simulink model "pfb_fft_vacc_sfloat_fft_dev"  (R2016b) (continued)
== system pfb_fft_vacc_sfloat_fft_dev == (continued)
      mask init:
      | pipeline_init(gcb,'latency',latency);
      settings: AttributesFormatString=Latency = %<latency>
      mask Display:
      | port_label('input', 1, 'd');
      | port_label('output', 1, 'q');
      | disp(['Z^-', num2str(latency)]);
  pipeline87 : Subsystem  AncestorBlock=casper_library_delays/pipeline  mask(pipeline){latency=1}
      mask help:
      | An explicitly laid-out delay line to avoid mapping to SRL16s
      | for use in pipelining to help achieve timing closure.
      mask init:
      | pipeline_init(gcb,'latency',latency);
      settings: AttributesFormatString=Latency = %<latency>
      mask Display:
      | port_label('input', 1, 'd');
      | port_label('output', 1, 'q');
      | disp(['Z^-', num2str(latency)]);
  pipeline88 : Subsystem  AncestorBlock=casper_library_delays/pipeline  mask(pipeline){latency=1}
      mask help:
      | An explicitly laid-out delay line to avoid mapping to SRL16s
      | for use in pipelining to help achieve timing closure.
      mask init:
      | pipeline_init(gcb,'latency',latency);
      settings: AttributesFormatString=Latency = %<latency>
      mask Display:
      | port_label('input', 1, 'd');
      | port_label('output', 1, 'q');
      | disp(['Z^-', num2str(latency)]);
  pipeline89 : Subsystem  AncestorBlock=casper_library_delays/pipeline  mask(pipeline){latency=1}
      mask help:
      | An explicitly laid-out delay line to avoid mapping to SRL16s
      | for use in pipelining to help achieve timing closure.
      mask init:
      | pipeline_init(gcb,'latency',latency);
      settings: AttributesFormatString=Latency = %<latency>
      mask Display:
      | port_label('input', 1, 'd');
      | port_label('output', 1, 'q');
      | disp(['Z^-', num2str(latency)]);
  pipeline9 : Subsystem  AncestorBlock=casper_library_delays/pipeline  mask(pipeline){latency=2}
      mask help:
      | An explicitly laid-out delay line to avoid mapping to SRL16s
      | for use in pipelining to help achieve timing closure.
      mask init:
      | pipeline_init(gcb,'latency',latency);
      settings: AttributesFormatString=Latency = %<latency>
      mask Display:
      | port_label('input', 1, 'd');
      | port_label('output', 1, 'q');
      | disp(['Z^-', num2str(latency)]);
  pipeline90 : Subsystem  AncestorBlock=casper_library_delays/pipeline  mask(pipeline){latency=2}
      mask help:
      | An explicitly laid-out delay line to avoid mapping to SRL16s
      | for use in pipelining to help achieve timing closure.
      mask init:
      | pipeline_init(gcb,'latency',latency);
      settings: AttributesFormatString=Latency = %<latency>
      mask Display:
      | port_label('input', 1, 'd');
      | port_label('output', 1, 'q');
      | disp(['Z^-', num2str(latency)]);
  pipeline91 : Subsystem  AncestorBlock=casper_library_delays/pipeline  mask(pipeline){latency=1}
      mask help:
      | An explicitly laid-out delay line to avoid mapping to SRL16s
      | for use in pipelining to help achieve timing closure.
      mask init:
      | pipeline_init(gcb,'latency',latency);
      settings: AttributesFormatString=Latency = %<latency>
      mask Display:
      | port_label('input', 1, 'd');
      | port_label('output', 1, 'q');
      | disp(['Z^-', num2str(latency)]);
  pipeline92 : Subsystem  AncestorBlock=casper_library_delays/pipeline  mask(pipeline){latency=1}
      mask help:
      | An explicitly laid-out delay line to avoid mapping to SRL16s
      | for use in pipelining to help achieve timing closure.
      mask init:
      | pipeline_init(gcb,'latency',latency);
      settings: AttributesFormatString=Latency = %<latency>
      mask Display:
      | port_label('input', 1, 'd');
      | port_label('output', 1, 'q');
      | disp(['Z^-', num2str(latency)]);
  pipeline93 : Subsystem  AncestorBlock=casper_library_delays/pipeline  mask(pipeline){latency=1}
      mask help:
      | An explicitly laid-out delay line to avoid mapping to SRL16s
      | for use in pipelining to help achieve timing closure.
      mask init:
      | pipeline_init(gcb,'latency',latency);
      settings: AttributesFormatString=Latency = %<latency>
      mask Display:
      | port_label('input', 1, 'd');
      | port_label('output', 1, 'q');
      | disp(['Z^-', num2str(latency)]);
  pipeline94 : Subsystem  AncestorBlock=casper_library_delays/pipeline  mask(pipeline){latency=2}
      mask help:
      | An explicitly laid-out delay line to avoid mapping to SRL16s
      | for use in pipelining to help achieve timing closure.
      mask init:
      | pipeline_init(gcb,'latency',latency);
      settings: AttributesFormatString=Latency = %<latency>
      mask Display:
      | port_label('input', 1, 'd');
      | port_label('output', 1, 'q');
      | disp(['Z^-', num2str(latency)]);
  pipeline95 : Subsystem  AncestorBlock=casper_library_delays/pipeline  mask(pipeline){latency=1}
      mask help:
      | An explicitly laid-out delay line to avoid mapping to SRL16s
      | for use in pipelining to help achieve timing closure.
      mask init:
      | pipeline_init(gcb,'latency',latency);
      settings: AttributesFormatString=Latency = %<latency>
      mask Display:
      | port_label('input', 1, 'd');
      | port_label('output', 1, 'q');
      | disp(['Z^-', num2str(latency)]);
  pipeline96 : Subsystem  AncestorBlock=casper_library_delays/pipeline  mask(pipeline){latency=2}
      mask help:
      | An explicitly laid-out delay line to avoid mapping to SRL16s
      | for use in pipelining to help achieve timing closure.
      mask init:
      | pipeline_init(gcb,'latency',latency);
      settings: AttributesFormatString=Latency = %<latency>
      mask Display:
      | port_label('input', 1, 'd');
      | port_label('output', 1, 'q');
      | disp(['Z^-', num2str(latency)]);
  pipeline97 : Subsystem  AncestorBlock=casper_library_delays/pipeline  mask(pipeline){latency=4}
      mask help:
      | An explicitly laid-out delay line to avoid mapping to SRL16s
      | for use in pipelining to help achieve timing closure.
      mask init:
      | pipeline_init(gcb,'latency',latency);
      settings: AttributesFormatString=Latency = %<latency>
      mask Display:
      | port_label('input', 1, 'd');
      | port_label('output', 1, 'q');
      | disp(['Z^-', num2str(latency)]);
  pipeline98 : Subsystem  AncestorBlock=casper_library_delays/pipeline  mask(pipeline){latency=2}
      mask help:
      | An explicitly laid-out delay line to avoid mapping to SRL16s
      | for use in pipelining to help achieve timing closure.
      mask init:
      | pipeline_init(gcb,'latency',latency);
      settings: AttributesFormatString=Latency = %<latency>
      mask Display:
      | port_label('input', 1, 'd');
      | port_label('output', 1, 'q');
      | disp(['Z^-', num2str(latency)]);
  pipeline99 : Subsystem  AncestorBlock=casper_library_delays/pipeline  mask(pipeline){latency=1}
      mask help:
      | An explicitly laid-out delay line to avoid mapping to SRL16s
      | for use in pipelining to help achieve timing closure.
      mask init:
      | pipeline_init(gcb,'latency',latency);
      settings: AttributesFormatString=Latency = %<latency>
      mask Display:
      | port_label('input', 1, 'd');
      | port_label('output', 1, 'q');
      | disp(['Z^-', num2str(latency)]);
  real_square{,1..3} (4 alike) : xbsIndex_r4/Mult  precision=Full  arith_type="Signed  (2's comp)"  n_bits=8  bin_pt=2  quantization=Truncate  overflow=Wrap  en=off  latency=6  use_behavioral_HDL=off  opt=Speed  use_embedded=on  optimum_pipeline=off  pipeline=on  dbl_ovrd=off  use_rpm=on  placement_style=Rectangular shape  block_type=mult
      settings: xl_area=[0, 0, 0, 0, 0, 0, 0]  block_version=9.1.01
  rpt_en_cwg0 : Subsystem  Tag=xps:sw_reg  AncestorBlock=xps_library/software_register  mask(swreg){io_dir=From Processor, io_delay=1, sample_period=1, names=[en dummy], bitwidths=[1 31], bin_pts=[0 0], arith_types=[0 0], sim_port=on, show_format=on}
      mask prompts: io_dir "I/O direction"; io_delay "I/O delay"; sample_period "Sample period"; names "Bitfield names [msb...lsb]"; bitwidths "Bitfield widths"; bin_pts "Bitfield binary pts"; arith_types "Bitfield types, ufix=0, fix=1, bool=2"; sim_port "Provide sim input/output?"; show_format "Print format string?"
      mask help: A 32-bit software-accessible register. Can be divided into bitfields of varying widths and types using the fields in the block mask.
      mask init:
      | swreg_init(gcb)
      settings: AttributesFormatString=2 inputs:uf1.0,uf31.0 = 32 bits
      mask TypeOptions io_dir: To Processor | From Processor
      mask Callback io_dir:
      | mask_enables = get_param(gcb, 'MaskEnables');
      | mask_names = get_param(gcb, 'MaskNames');
      | if strcmp(get_param(gcb, 'io_dir'), 'To Processor'),
      |     mask_enables{ismember(mask_names, 'sample_period')} = 'off';
      | else
      |     mask_enables{ismember(mask_names, 'sample_period')} = 'on';
      | end
      | set_param(gcb, 'MaskEnables', mask_enables);
  rpt_length_cwg0 : Subsystem  Tag=xps:sw_reg  AncestorBlock=xps_library/software_register  mask(swreg){io_dir=From Processor, io_delay=1, sample_period=1, names=cwg0_repeat_length, bitwidths=32, bin_pts=0, arith_types=0, sim_port=on, show_format=on}
      mask prompts: io_dir "I/O direction"; io_delay "I/O delay"; sample_period "Sample period"; names "Bitfield names [msb...lsb]"; bitwidths "Bitfield widths"; bin_pts "Bitfield binary pts"; arith_types "Bitfield types, ufix=0, fix=1, bool=2"; sim_port "Provide sim input/output?"; show_format "Print format string?"
      mask help: A 32-bit software-accessible register. Can be divided into bitfields of varying widths and types using the fields in the block mask.
      mask init:
      | swreg_init(gcb)
      settings: AttributesFormatString=1input:uf32.0 = 32 bits
      mask TypeOptions io_dir: To Processor | From Processor
      mask Callback io_dir:
      | mask_enables = get_param(gcb, 'MaskEnables');
      | mask_names = get_param(gcb, 'MaskNames');
      | if strcmp(get_param(gcb, 'io_dir'), 'To Processor'),
      |     mask_enables{ismember(mask_names, 'sample_period')} = 'off';
      | else
      |     mask_enables{ismember(mask_names, 'sample_period')} = 'on';
      | end
      | set_param(gcb, 'MaskEnables', mask_enables);
  scale_cwg0 : Subsystem  Tag=xps:sw_reg  AncestorBlock=xps_library/software_register  mask(swreg){io_dir=From Processor, io_delay=1, sample_period=1, names=[scale dummy], bitwidths=16, bin_pts=15, arith_types=0, sim_port=on, show_format=on}
      mask prompts: io_dir "I/O direction"; io_delay "I/O delay"; sample_period "Sample period"; names "Bitfield names [msb...lsb]"; bitwidths "Bitfield widths"; bin_pts "Bitfield binary pts"; arith_types "Bitfield types, ufix=0, fix=1, bool=2"; sim_port "Provide sim input/output?"; show_format "Print format string?"
      mask help: A 32-bit software-accessible register. Can be divided into bitfields of varying widths and types using the fields in the block mask.
      mask init:
      | swreg_init(gcb)
      settings: AttributesFormatString=2 inputs:uf16.15,uf16.15 = 32 bits
      mask TypeOptions io_dir: To Processor | From Processor
      mask Callback io_dir:
      | mask_enables = get_param(gcb, 'MaskEnables');
      | mask_names = get_param(gcb, 'MaskNames');
      | if strcmp(get_param(gcb, 'io_dir'), 'To Processor'),
      |     mask_enables{ismember(mask_names, 'sample_period')} = 'off';
      | else
      |     mask_enables{ismember(mask_names, 'sample_period')} = 'on';
      | end
      | set_param(gcb, 'MaskEnables', mask_enables);
  scale_float : Subsystem
  scale_float1 : Subsystem
  scale_float2 : Subsystem
  scale_float3 : Subsystem
  scale_out0 : Subsystem  Tag=xps:sw_reg  AncestorBlock=xps_library/software_register  mask(swreg){io_dir=From Processor, io_delay=1, sample_period=1, names=[scale dummy], bitwidths=16, bin_pts=15, arith_types=0, sim_port=on, show_format=on}
      mask prompts: io_dir "I/O direction"; io_delay "I/O delay"; sample_period "Sample period"; names "Bitfield names [msb...lsb]"; bitwidths "Bitfield widths"; bin_pts "Bitfield binary pts"; arith_types "Bitfield types, ufix=0, fix=1, bool=2"; sim_port "Provide sim input/output?"; show_format "Print format string?"
      mask help: A 32-bit software-accessible register. Can be divided into bitfields of varying widths and types using the fields in the block mask.
      mask init:
      | swreg_init(gcb)
      settings: AttributesFormatString=2 inputs:uf16.15,uf16.15 = 32 bits
      mask TypeOptions io_dir: To Processor | From Processor
      mask Callback io_dir:
      | mask_enables = get_param(gcb, 'MaskEnables');
      | mask_names = get_param(gcb, 'MaskNames');
      | if strcmp(get_param(gcb, 'io_dir'), 'To Processor'),
      |     mask_enables{ismember(mask_names, 'sample_period')} = 'off';
      | else
      |     mask_enables{ismember(mask_names, 'sample_period')} = 'on';
      | end
      | set_param(gcb, 'MaskEnables', mask_enables);
  scale_wng0 : Subsystem  Tag=xps:sw_reg  AncestorBlock=xps_library/software_register  mask(swreg){io_dir=From Processor, io_delay=1, sample_period=1, names=[scale dummy], bitwidths=16, bin_pts=15, arith_types=0, sim_port=on, show_format=on}
      mask prompts: io_dir "I/O direction"; io_delay "I/O delay"; sample_period "Sample period"; names "Bitfield names [msb...lsb]"; bitwidths "Bitfield widths"; bin_pts "Bitfield binary pts"; arith_types "Bitfield types, ufix=0, fix=1, bool=2"; sim_port "Provide sim input/output?"; show_format "Print format string?"
      mask help: A 32-bit software-accessible register. Can be divided into bitfields of varying widths and types using the fields in the block mask.
      mask init:
      | swreg_init(gcb)
      settings: AttributesFormatString=2 inputs:uf16.15,uf16.15 = 32 bits
      mask TypeOptions io_dir: To Processor | From Processor
      mask Callback io_dir:
      | mask_enables = get_param(gcb, 'MaskEnables');
      | mask_names = get_param(gcb, 'MaskNames');
      | if strcmp(get_param(gcb, 'io_dir'), 'To Processor'),
      |     mask_enables{ismember(mask_names, 'sample_period')} = 'off';
      | else
      |     mask_enables{ismember(mask_names, 'sample_period')} = 'on';
      | end
      | set_param(gcb, 'MaskEnables', mask_enables);
  simple_bram_vacc : Subsystem  AncestorBlock=casper_library_accumulators/simple_bram_vacc  mask(simple_bram_vacc){vec_len=2^10, arith_type=Signed, n_bits=32, bin_pt=7}
      mask prompts: vec_len "Vector length:"; arith_type "Output type:"; n_bits "Bit width (output):"; bin_pt "Binary point (output):"
      mask help: This simple vector accumulator is based on a shift register. It outputs its previous accumulated vector after a new_acc pulse is received.
      mask init:
      | simple_bram_vacc_init(gcb, ...
      |     'vec_len', vec_len, ...
      |     'arith_type', arith_type, ...
      |     'n_bits', n_bits, ...
      |     'bin_pt', bin_pt);
      mask TypeOptions arith_type: Signed | Unsigned
  simple_bram_vacc1 : Subsystem  AncestorBlock=casper_library_accumulators/simple_bram_vacc  mask(simple_bram_vacc){vec_len=2^10, arith_type=Signed, n_bits=32, bin_pt=7}
      mask prompts: vec_len "Vector length:"; arith_type "Output type:"; n_bits "Bit width (output):"; bin_pt "Binary point (output):"
      mask help: This simple vector accumulator is based on a shift register. It outputs its previous accumulated vector after a new_acc pulse is received.
      mask init:
      | simple_bram_vacc_init(gcb, ...
      |     'vec_len', vec_len, ...
      |     'arith_type', arith_type, ...
      |     'n_bits', n_bits, ...
      |     'bin_pt', bin_pt);
      mask TypeOptions arith_type: Signed | Unsigned
  simple_bram_vacc2 : Subsystem  AncestorBlock=casper_library_accumulators/simple_bram_vacc  mask(simple_bram_vacc){vec_len=2^10, arith_type=Signed, n_bits=32, bin_pt=7}
      mask prompts: vec_len "Vector length:"; arith_type "Output type:"; n_bits "Bit width (output):"; bin_pt "Binary point (output):"
      mask help: This simple vector accumulator is based on a shift register. It outputs its previous accumulated vector after a new_acc pulse is received.
      mask init:
      | simple_bram_vacc_init(gcb, ...
      |     'vec_len', vec_len, ...
      |     'arith_type', arith_type, ...
      |     'n_bits', n_bits, ...
      |     'bin_pt', bin_pt);
      mask TypeOptions arith_type: Signed | Unsigned
  simple_bram_vacc3 : Subsystem  AncestorBlock=casper_library_accumulators/simple_bram_vacc  mask(simple_bram_vacc){vec_len=2^10, arith_type=Signed, n_bits=32, bin_pt=7}
      mask prompts: vec_len "Vector length:"; arith_type "Output type:"; n_bits "Bit width (output):"; bin_pt "Binary point (output):"
      mask help: This simple vector accumulator is based on a shift register. It outputs its previous accumulated vector after a new_acc pulse is received.
      mask init:
      | simple_bram_vacc_init(gcb, ...
      |     'vec_len', vec_len, ...
      |     'arith_type', arith_type, ...
      |     'n_bits', n_bits, ...
      |     'bin_pt', bin_pt);
      mask TypeOptions arith_type: Signed | Unsigned
  simple_bram_vacc_float1 : Subsystem  AncestorBlock=casper_library_accumulators/simple_bram_vacc  mask(simple_bram_vacc){vec_len=2^10, arith_type=Unsigned, n_bits=128, bin_pt=68}
      mask prompts: vec_len "Vector length:"; arith_type "Output type:"; n_bits "Bit width (output):"; bin_pt "Binary point (output):"
      mask help: This simple vector accumulator is based on a shift register. It outputs its previous accumulated vector after a new_acc pulse is received.
      mask init:
      | % simple_bram_vacc_init(gcb, ...
      | %     'vec_len', vec_len, ...
      | %     'arith_type', arith_type, ...
      | %     'n_bits', n_bits, ...
      | %     'bin_pt', bin_pt);
      mask TypeOptions arith_type: Signed | Unsigned
  spect_cnt : xbsIndex_r4/Counter  cnt_type=Free Running  cnt_to=Inf  operation=Up  start_count=0  cnt_by_val=1  arith_type=Unsigned  n_bits=32  bin_pt=0  load_pin=off  rst=on  en=on  explicit_period=on  period=1  use_behavioral_HDL=off  implementation=Fabric  use_rpm=off  dbl_ovrd=off  block_type=counter
  spectrum_counter : Subsystem  Tag=xps:sw_reg  AncestorBlock=xps_library/software_register  mask(swreg){io_dir=To Processor, io_delay=0, sample_period=1, names=reg, bitwidths=32, bin_pts=0, arith_types=0, sim_port=on, show_format=on}
      mask prompts: io_dir "I/O direction"; io_delay "I/O delay"; sample_period "Sample period"; names "Bitfield names [msb...lsb]"; bitwidths "Bitfield widths"; bin_pts "Bitfield binary pts"; arith_types "Bitfield types, ufix=0, fix=1, bool=2"; sim_port "Provide sim input/output?"; show_format "Print format string?"
      mask help: A 32-bit software-accessible register. Can be divided into bitfields of varying widths and types using the fields in the block mask.
      mask init:
      | swreg_init(gcb)
      settings: AttributesFormatString=1output:uf32.0 = 32 bits
      mask TypeOptions io_dir: To Processor | From Processor
      mask Callback io_dir:
      | mask_enables = get_param(gcb, 'MaskEnables');
      | mask_names = get_param(gcb, 'MaskNames');
      | if strcmp(get_param(gcb, 'io_dir'), 'To Processor'),
      |     mask_enables{ismember(mask_names, 'sample_period')} = 'off';
      | else
      |     mask_enables{ismember(mask_names, 'sample_period')} = 'on';
      | end
      | set_param(gcb, 'MaskEnables', mask_enables);
  spectrum_limit : Subsystem  Tag=xps:sw_reg  AncestorBlock=xps_library/software_register  mask(swreg){io_dir=From Processor, io_delay=0, sample_period=1, names=limit, bitwidths=32, bin_pts=0, arith_types=0, sim_port=on, show_format=on}
      mask prompts: io_dir "I/O direction"; io_delay "I/O delay"; sample_period "Sample period"; names "Bitfield names [msb...lsb]"; bitwidths "Bitfield widths"; bin_pts "Bitfield binary pts"; arith_types "Bitfield types, ufix=0, fix=1, bool=2"; sim_port "Provide sim input/output?"; show_format "Print format string?"
      mask help: A 32-bit software-accessible register. Can be divided into bitfields of varying widths and types using the fields in the block mask.
      mask init:
      | swreg_init(gcb)
      settings: AttributesFormatString=1input:uf32.0 = 32 bits
      mask TypeOptions io_dir: To Processor | From Processor
      mask Callback io_dir:
      | mask_enables = get_param(gcb, 'MaskEnables');
      | mask_names = get_param(gcb, 'MaskNames');
      | if strcmp(get_param(gcb, 'io_dir'), 'To Processor'),
      |     mask_enables{ismember(mask_names, 'sample_period')} = 'off';
      | else
      |     mask_enables{ismember(mask_names, 'sample_period')} = 'on';
      | end
      | set_param(gcb, 'MaskEnables', mask_enables);
  ss_pfb_raw : Subsystem  Tag=casper:bitsnap  AncestorBlock=casper_library_scopes/bitfield_snapshot  mask(bitsnap){io_names=[r0 i0 r1 i1 acc], io_widths=[18 18 18 18 32], io_bps=[17 17 17 17 0], io_types=[1 1 1 1 0], show_format=on, snap_storage=bram, snap_dram_dimm=2, snap_dram_clock=250, snap_nsamples=10, snap_data_width=128, snap_offset=off, snap_circap=off, snap_value=off, snap_ext_arm=off, snap_provide_outputs=on, snap_use_dsp48=on, snap_delay=2, extra_names=[c d], extra_widths=[30 2], extra_bps=[0 0], extra_types=[0 0]}
      mask prompts: io_names "Names [msb...lsb]"; io_widths "Bitwidths [msb...lsb]"; io_bps "Binary pts [msb...lsb]"; io_types "Data types [msb...lsb], ufix=0, fix=1, bool=2"; show_format "Print format string?"; snap_storage "Storage medium"; snap_dram_dimm "DRAM dimm"; snap_dram_clock "DRAM clock rate"; snap_nsamples "Number of Samples (2^?)"; snap_data_width "Data width"; snap_offset "Start delay support"; snap_circap "Circular capture support"; snap_value "Extra value capture support"; snap_ext_arm "External arm support"; snap_provide_outputs "Provide outputs"; snap_use_dsp48 "Use DSP48s to implement counters"; snap_delay "Pre-snapshot delay"; extra_names "Names [msb...lsb]"; extra_widths "Widths [msb...lsb]"; extra_bps "Binary pts [msb...lsb]"; extra_types "Data types [msb...lsb], ufix=0, fix=1, bool=2"
      mask help:
      | A wrapped snapshot block. Allows specification of inputs to a snapblock word so the toolflow can automatically set up the scope.
      | See documentation for snapshot for explanation of parameters.
      mask init:
      | bitsnap_init(gcb);
      settings: AttributesFormatString=|
      | snap(5):f18.17,f18.17,f18.17,f18.17,uf32.0 = 104 bits\n128 wide, 1024 deep
      | debugID: 130880866
      mask TypeOptions snap_storage: bram | dram
      mask Callback snap_storage: bitsnap_callback();
      mask TypeOptions snap_dram_dimm: 1 | 2 | 3 | 4
      mask TypeOptions snap_dram_clock: 200 | 250 | 266 | 300 | 333
      mask TypeOptions snap_data_width: 8 | 16 | 32 | 64 | 128
      mask Callback snap_value: bitsnap_callback();
      mask Callback snap_ext_arm: bitsnap_callback();
      mask Callback snap_provide_outputs: bitsnap_callback();
  ss_pfb_raw1 : Subsystem  Tag=casper:bitsnap  AncestorBlock=casper_library_scopes/bitfield_snapshot  mask(bitsnap){io_names=[r2 i2 r3 i3], io_widths=[18 18 18 18], io_bps=[17 17 17 17], io_types=[1 1 1 1], show_format=on, snap_storage=bram, snap_dram_dimm=2, snap_dram_clock=250, snap_nsamples=10, snap_data_width=128, snap_offset=off, snap_circap=off, snap_value=off, snap_ext_arm=off, snap_provide_outputs=on, snap_use_dsp48=on, snap_delay=2, extra_names=[c d], extra_widths=[30 2], extra_bps=[0 0], extra_types=[0 0]}
      mask prompts: io_names "Names [msb...lsb]"; io_widths "Bitwidths [msb...lsb]"; io_bps "Binary pts [msb...lsb]"; io_types "Data types [msb...lsb], ufix=0, fix=1, bool=2"; show_format "Print format string?"; snap_storage "Storage medium"; snap_dram_dimm "DRAM dimm"; snap_dram_clock "DRAM clock rate"; snap_nsamples "Number of Samples (2^?)"; snap_data_width "Data width"; snap_offset "Start delay support"; snap_circap "Circular capture support"; snap_value "Extra value capture support"; snap_ext_arm "External arm support"; snap_provide_outputs "Provide outputs"; snap_use_dsp48 "Use DSP48s to implement counters"; snap_delay "Pre-snapshot delay"; extra_names "Names [msb...lsb]"; extra_widths "Widths [msb...lsb]"; extra_bps "Binary pts [msb...lsb]"; extra_types "Data types [msb...lsb], ufix=0, fix=1, bool=2"
      mask help:
      | A wrapped snapshot block. Allows specification of inputs to a snapblock word so the toolflow can automatically set up the scope.
      | See documentation for snapshot for explanation of parameters.
      mask init:
      | bitsnap_init(gcb);
      settings: AttributesFormatString=|
      | snap(4):f18.17,f18.17,f18.17,f18.17 = 72 bits\n128 wide, 1024 deep
      | debugID: 30769355
      mask TypeOptions snap_storage: bram | dram
      mask Callback snap_storage: bitsnap_callback();
      mask TypeOptions snap_dram_dimm: 1 | 2 | 3 | 4
      mask TypeOptions snap_dram_clock: 200 | 250 | 266 | 300 | 333
      mask TypeOptions snap_data_width: 8 | 16 | 32 | 64 | 128
      mask Callback snap_value: bitsnap_callback();
      mask Callback snap_ext_arm: bitsnap_callback();
      mask Callback snap_provide_outputs: bitsnap_callback();
  ss_pfb_sq0 : Subsystem  Tag=casper:bitsnap  AncestorBlock=casper_library_scopes/bitfield_snapshot  mask(bitsnap){io_names=[pfb2_0], io_widths=[32], io_bps=[7], io_types=[1], show_format=on, snap_storage=bram, snap_dram_dimm=2, snap_dram_clock=250, snap_nsamples=10, snap_data_width=32, snap_offset=off, snap_circap=off, snap_value=off, snap_ext_arm=off, snap_provide_outputs=on, snap_use_dsp48=on, snap_delay=2, extra_names=[c d], extra_widths=[30 2], extra_bps=[0 0], extra_types=[0 0]}
      mask prompts: io_names "Names [msb...lsb]"; io_widths "Bitwidths [msb...lsb]"; io_bps "Binary pts [msb...lsb]"; io_types "Data types [msb...lsb], ufix=0, fix=1, bool=2"; show_format "Print format string?"; snap_storage "Storage medium"; snap_dram_dimm "DRAM dimm"; snap_dram_clock "DRAM clock rate"; snap_nsamples "Number of Samples (2^?)"; snap_data_width "Data width"; snap_offset "Start delay support"; snap_circap "Circular capture support"; snap_value "Extra value capture support"; snap_ext_arm "External arm support"; snap_provide_outputs "Provide outputs"; snap_use_dsp48 "Use DSP48s to implement counters"; snap_delay "Pre-snapshot delay"; extra_names "Names [msb...lsb]"; extra_widths "Widths [msb...lsb]"; extra_bps "Binary pts [msb...lsb]"; extra_types "Data types [msb...lsb], ufix=0, fix=1, bool=2"
      mask help:
      | A wrapped snapshot block. Allows specification of inputs to a snapblock word so the toolflow can automatically set up the scope.
      | See documentation for snapshot for explanation of parameters.
      mask init:
      | bitsnap_init(gcb);
      settings: AttributesFormatString=|
      | snap(1):f32.7 = 32 bits\n32 wide, 1024 deep
      | debugID: 37953464
      mask TypeOptions snap_storage: bram | dram
      mask Callback snap_storage: bitsnap_callback();
      mask TypeOptions snap_dram_dimm: 1 | 2 | 3 | 4
      mask TypeOptions snap_dram_clock: 200 | 250 | 266 | 300 | 333
      mask TypeOptions snap_data_width: 8 | 16 | 32 | 64 | 128
      mask Callback snap_value: bitsnap_callback();
      mask Callback snap_ext_arm: bitsnap_callback();
      mask Callback snap_provide_outputs: bitsnap_callback();
  ss_pfb_sq1 : Subsystem  Tag=casper:bitsnap  AncestorBlock=casper_library_scopes/bitfield_snapshot  mask(bitsnap){io_names=[pfb2_0], io_widths=[32], io_bps=[7], io_types=[1], show_format=on, snap_storage=bram, snap_dram_dimm=2, snap_dram_clock=250, snap_nsamples=10, snap_data_width=32, snap_offset=off, snap_circap=off, snap_value=off, snap_ext_arm=off, snap_provide_outputs=on, snap_use_dsp48=on, snap_delay=2, extra_names=[c d], extra_widths=[30 2], extra_bps=[0 0], extra_types=[0 0]}
      mask prompts: io_names "Names [msb...lsb]"; io_widths "Bitwidths [msb...lsb]"; io_bps "Binary pts [msb...lsb]"; io_types "Data types [msb...lsb], ufix=0, fix=1, bool=2"; show_format "Print format string?"; snap_storage "Storage medium"; snap_dram_dimm "DRAM dimm"; snap_dram_clock "DRAM clock rate"; snap_nsamples "Number of Samples (2^?)"; snap_data_width "Data width"; snap_offset "Start delay support"; snap_circap "Circular capture support"; snap_value "Extra value capture support"; snap_ext_arm "External arm support"; snap_provide_outputs "Provide outputs"; snap_use_dsp48 "Use DSP48s to implement counters"; snap_delay "Pre-snapshot delay"; extra_names "Names [msb...lsb]"; extra_widths "Widths [msb...lsb]"; extra_bps "Binary pts [msb...lsb]"; extra_types "Data types [msb...lsb], ufix=0, fix=1, bool=2"
      mask help:
      | A wrapped snapshot block. Allows specification of inputs to a snapblock word so the toolflow can automatically set up the scope.
      | See documentation for snapshot for explanation of parameters.
      mask init:
      | bitsnap_init(gcb);
      settings: AttributesFormatString=|
      | snap(1):f32.7 = 32 bits\n32 wide, 1024 deep
      | debugID: 37983255
      mask TypeOptions snap_storage: bram | dram
      mask Callback snap_storage: bitsnap_callback();
      mask TypeOptions snap_dram_dimm: 1 | 2 | 3 | 4
      mask TypeOptions snap_dram_clock: 200 | 250 | 266 | 300 | 333
      mask TypeOptions snap_data_width: 8 | 16 | 32 | 64 | 128
      mask Callback snap_value: bitsnap_callback();
      mask Callback snap_ext_arm: bitsnap_callback();
      mask Callback snap_provide_outputs: bitsnap_callback();
  ss_pfb_sq2 : Subsystem  Tag=casper:bitsnap  AncestorBlock=casper_library_scopes/bitfield_snapshot  mask(bitsnap){io_names=[pfb2_0], io_widths=[32], io_bps=[7], io_types=[1], show_format=on, snap_storage=bram, snap_dram_dimm=2, snap_dram_clock=250, snap_nsamples=10, snap_data_width=32, snap_offset=off, snap_circap=off, snap_value=off, snap_ext_arm=off, snap_provide_outputs=on, snap_use_dsp48=on, snap_delay=2, extra_names=[c d], extra_widths=[30 2], extra_bps=[0 0], extra_types=[0 0]}
      mask prompts: io_names "Names [msb...lsb]"; io_widths "Bitwidths [msb...lsb]"; io_bps "Binary pts [msb...lsb]"; io_types "Data types [msb...lsb], ufix=0, fix=1, bool=2"; show_format "Print format string?"; snap_storage "Storage medium"; snap_dram_dimm "DRAM dimm"; snap_dram_clock "DRAM clock rate"; snap_nsamples "Number of Samples (2^?)"; snap_data_width "Data width"; snap_offset "Start delay support"; snap_circap "Circular capture support"; snap_value "Extra value capture support"; snap_ext_arm "External arm support"; snap_provide_outputs "Provide outputs"; snap_use_dsp48 "Use DSP48s to implement counters"; snap_delay "Pre-snapshot delay"; extra_names "Names [msb...lsb]"; extra_widths "Widths [msb...lsb]"; extra_bps "Binary pts [msb...lsb]"; extra_types "Data types [msb...lsb], ufix=0, fix=1, bool=2"
      mask help:
      | A wrapped snapshot block. Allows specification of inputs to a snapblock word so the toolflow can automatically set up the scope.
      | See documentation for snapshot for explanation of parameters.
      mask init:
      | bitsnap_init(gcb);
      settings: AttributesFormatString=|
      | snap(1):f32.7 = 32 bits\n32 wide, 1024 deep
      | debugID: 38013046
      mask TypeOptions snap_storage: bram | dram
      mask Callback snap_storage: bitsnap_callback();
      mask TypeOptions snap_dram_dimm: 1 | 2 | 3 | 4
      mask TypeOptions snap_dram_clock: 200 | 250 | 266 | 300 | 333
      mask TypeOptions snap_data_width: 8 | 16 | 32 | 64 | 128
      mask Callback snap_value: bitsnap_callback();
      mask Callback snap_ext_arm: bitsnap_callback();
      mask Callback snap_provide_outputs: bitsnap_callback();
  ss_pfb_sq3 : Subsystem  Tag=casper:bitsnap  AncestorBlock=casper_library_scopes/bitfield_snapshot  mask(bitsnap){io_names=[pfb2_0], io_widths=[32], io_bps=[7], io_types=[1], show_format=on, snap_storage=bram, snap_dram_dimm=2, snap_dram_clock=250, snap_nsamples=10, snap_data_width=32, snap_offset=off, snap_circap=off, snap_value=off, snap_ext_arm=off, snap_provide_outputs=on, snap_use_dsp48=on, snap_delay=2, extra_names=[c d], extra_widths=[30 2], extra_bps=[0 0], extra_types=[0 0]}
      mask prompts: io_names "Names [msb...lsb]"; io_widths "Bitwidths [msb...lsb]"; io_bps "Binary pts [msb...lsb]"; io_types "Data types [msb...lsb], ufix=0, fix=1, bool=2"; show_format "Print format string?"; snap_storage "Storage medium"; snap_dram_dimm "DRAM dimm"; snap_dram_clock "DRAM clock rate"; snap_nsamples "Number of Samples (2^?)"; snap_data_width "Data width"; snap_offset "Start delay support"; snap_circap "Circular capture support"; snap_value "Extra value capture support"; snap_ext_arm "External arm support"; snap_provide_outputs "Provide outputs"; snap_use_dsp48 "Use DSP48s to implement counters"; snap_delay "Pre-snapshot delay"; extra_names "Names [msb...lsb]"; extra_widths "Widths [msb...lsb]"; extra_bps "Binary pts [msb...lsb]"; extra_types "Data types [msb...lsb], ufix=0, fix=1, bool=2"
      mask help:
      | A wrapped snapshot block. Allows specification of inputs to a snapblock word so the toolflow can automatically set up the scope.
      | See documentation for snapshot for explanation of parameters.
      mask init:
      | bitsnap_init(gcb);
      settings: AttributesFormatString=|
      | snap(1):f32.7 = 32 bits\n32 wide, 1024 deep
      | debugID: 38042837
      mask TypeOptions snap_storage: bram | dram
      mask Callback snap_storage: bitsnap_callback();
      mask TypeOptions snap_dram_dimm: 1 | 2 | 3 | 4
      mask TypeOptions snap_dram_clock: 200 | 250 | 266 | 300 | 333
      mask TypeOptions snap_data_width: 8 | 16 | 32 | 64 | 128
      mask Callback snap_value: bitsnap_callback();
      mask Callback snap_ext_arm: bitsnap_callback();
      mask Callback snap_provide_outputs: bitsnap_callback();
  sys_en : Subsystem  Tag=xps:sw_reg  AncestorBlock=xps_library/software_register  mask(swreg){io_dir=From Processor, io_delay=0, sample_period=1, names=en, bitwidths=1, bin_pts=0, arith_types=2, sim_port=on, show_format=on}
      mask prompts: io_dir "I/O direction"; io_delay "I/O delay"; sample_period "Sample period"; names "Bitfield names [msb...lsb]"; bitwidths "Bitfield widths"; bin_pts "Bitfield binary pts"; arith_types "Bitfield types, ufix=0, fix=1, bool=2"; sim_port "Provide sim input/output?"; show_format "Print format string?"
      mask help: A 32-bit software-accessible register. Can be divided into bitfields of varying widths and types using the fields in the block mask.
      mask init:
      | swreg_init(gcb)
      settings: AttributesFormatString=1input:b = 1 bits
      mask TypeOptions io_dir: To Processor | From Processor
      mask Callback io_dir:
      | mask_enables = get_param(gcb, 'MaskEnables');
      | mask_names = get_param(gcb, 'MaskNames');
      | if strcmp(get_param(gcb, 'io_dir'), 'To Processor'),
      |     mask_enables{ismember(mask_names, 'sample_period')} = 'off';
      | else
      |     mask_enables{ismember(mask_names, 'sample_period')} = 'on';
      | end
      | set_param(gcb, 'MaskEnables', mask_enables);
  sys_rst : Subsystem  Tag=xps:sw_reg  AncestorBlock=xps_library/software_register  mask(swreg){io_dir=From Processor, io_delay=0, sample_period=1, names=rst, bitwidths=1, bin_pts=0, arith_types=2, sim_port=on, show_format=on}
      mask prompts: io_dir "I/O direction"; io_delay "I/O delay"; sample_period "Sample period"; names "Bitfield names [msb...lsb]"; bitwidths "Bitfield widths"; bin_pts "Bitfield binary pts"; arith_types "Bitfield types, ufix=0, fix=1, bool=2"; sim_port "Provide sim input/output?"; show_format "Print format string?"
      mask help: A 32-bit software-accessible register. Can be divided into bitfields of varying widths and types using the fields in the block mask.
      mask init:
      | swreg_init(gcb)
      settings: AttributesFormatString=1input:b = 1 bits
      mask TypeOptions io_dir: To Processor | From Processor
      mask Callback io_dir:
      | mask_enables = get_param(gcb, 'MaskEnables');
      | mask_names = get_param(gcb, 'MaskNames');
      | if strcmp(get_param(gcb, 'io_dir'), 'To Processor'),
      |     mask_enables{ismember(mask_names, 'sample_period')} = 'off';
      | else
      |     mask_enables{ismember(mask_names, 'sample_period')} = 'on';
      | end
      | set_param(gcb, 'MaskEnables', mask_enables);
  tvg_sel : Subsystem  Tag=xps:sw_reg  AncestorBlock=xps_library/software register  mask(swreg){io_dir=From Processor, io_delay=0, sample_period=1, names=[tvg_sel], bitwidths=[1], bin_pts=0, arith_types=[0], sim_port=on, show_format=on}
      mask prompts: io_dir "I/O direction"; io_delay "I/O delay"; sample_period "Sample period"; names "Bitfield names [msb...lsb]"; bitwidths "Bitfield widths"; bin_pts "Bitfield binary pts"; arith_types "Bitfield types, ufix=0, fix=1, bool=2"; sim_port "Provide sim input/output?"; show_format "Print format string?"
      mask help: A 32-bit software-accessible register. Can be divided into bitfields of varying widths and types using the fields in the block mask.
      mask init:
      | swreg_init(gcb)
      settings: AttributesFormatString=1input:uf1.0 = 1 bits
      mask TypeOptions io_dir: To Processor | From Processor
      mask Callback io_dir:
      | mask_enables = get_param(gcb, 'MaskEnables');
      | mask_names = get_param(gcb, 'MaskNames');
      | if strcmp(get_param(gcb, 'io_dir'), 'To Processor'),
      |     mask_enables{ismember(mask_names, 'sample_period')} = 'off';
      | else
      |     mask_enables{ismember(mask_names, 'sample_period')} = 'on';
      | end
      | set_param(gcb, 'MaskEnables', mask_enables);
  wng0 : Subsystem
  tag scope: acc_len=global, acc_scale=global, cnt_rst=global
  wires:
  pfb/2(dout0) -> pipeline81/1(d)
  pfb/3(dout1) -> pipeline82/1(d)
  [fft_shift] -> pfb/1(shift)
  pfb/5(of) -> pipeline84/1(d)
  pfb/4(dvalid) -> pipeline83/1(d)
  pfb/1(sync_out) -> pipeline80/1(d)
  [pfbin_d1] -> pfb/4(din1)
  [pfbin_sync] -> pfb/2(sync_in)
  [pfbin_dv] -> pfb/5(en)
  fft_shift/1(in_fft_shift) -> [fft_shift]
  [pfb_dfloat_1] -> Terminator2/1
  Gateway Out8/1 -> To Workspace1/1
  [pfb_sync] -> Gateway Out8/1
  Gateway Out9/1 -> To Workspace10/1
  [pfb_dv] -> Gateway Out9/1
  edge_detect/1 -> pipeline1/1(d)
  pipeline1/1(q) -> [pfbin_sync]
  pipeline2/1(q) -> [pfbin_d0]
  pipeline3/1(q) -> [pfbin_d1]
  pipeline5/1(q) -> [pfbin_dv]
  Constant3/1 -> fft_shift/1(sim_1)
  Constant2/1 -> pipeline3/1(d)
  Counter/1 -> Relational/1
  Constant7/1 -> Relational/2
  Relational/1 -> Register/1, Convert/1, Register/3
  [pfbin_d0] -> pfb/3(din0)
  Register/1 -> pipeline79/1(d)
  Constant8/1 -> Concat/1
  Convert/1 -> Concat1/1
  Constant9/1 -> Concat1/2
  Mux/1 -> pipeline2/1(d)
  Gateway Out18/1 -> To Workspace42/1
  [pfbin_sync] -> Gateway Out18/1
  Gateway Out41/1 -> To Workspace44/1
  [pfbin_dv] -> Gateway Out41/1
  Gateway Out51/1 -> To Workspace3/1
  [pfb_of] -> Gateway Out51/1
  Counter3/1 -> Relational1/1
  Constant/1 -> Relational1/2
  [local_rst] -> edge_detect5/1
  edge_detect5/1 -> Counter7/1
  spectrum_counter/1(sim_out) -> Terminator16/1
  Constant48/1 -> spectrum_limit/1(sim_1)
  spectrum_limit/1(in_limit) -> [spec_limit], Gateway Out89/1
  Counter7/1 -> [spec_counter], Gateway Out88/1
  [spec_counter] -> pipeline6/1(d)
  Gateway Out88/1 -> To Workspace88/1
  Gateway Out89/1 -> To Workspace89/1
  Relational1/1 -> edge_detect2/1
  [local_rst] -> edge_detect3/1
  [spec_trig] -> Counter7/2
  Constant4/1 -> sys_rst/1(sim_1)
  sys_rst/1(in_rst) -> pipeline8/1(d)
  pipeline8/1(q) -> edge_detect1/1
  [rst] -> Register/2, Counter/1
  edge_detect1/1 -> [rst]
  [rst] -> Counter4/1
  Counter4/1 -> pipeline9/1(d)
  pfbin_sync_count/1(sim_out) -> Terminator17/1
  pipeline9/1(q) -> pfbin_sync_count/1(out_reg), Gateway Out69/1
  Gateway Out69/1 -> To Workspace69/1
  [pfbin_sync] -> Counter4/2
  [rst] -> Counter5/1
  Counter5/1 -> pipeline10/1(d)
  pfbout_sync_count/1(sim_out) -> Terminator18/1
  pipeline10/1(q) -> Gateway Out70/1, pfbout_sync_count/1(out_reg)
  Gateway Out70/1 -> To Workspace70/1
  [pfb_sync] -> Counter5/2
  edge_detect3/1 -> Counter3/1
  [rst] -> forty_gbe/1(rst)
  Constant18/1 -> forty_gbe/7(rx_ack)
  Constant19/1 -> forty_gbe/8(rx_overrun_ack)
  Constant20/1 -> forty_gbe/9(debug_rst)
  Constant21/1 -> forty_gbe/6(tx_end_of_frame)
  Constant46/1 -> forty_gbe/3(tx_valid)
  Constant47/1 -> forty_gbe/2(tx_data)
  Constant49/1 -> forty_gbe/4(tx_dest_ip)
  Constant50/1 -> forty_gbe/5(tx_dest_port)
  edge_detect2/1 -> [spec_trig]
  Constant1/1 -> tvg_sel/1(sim_1)
  Gateway Out44/1 -> To Workspace72/1
  Gateway Out47/1 -> To Workspace73/1
  Gateway Out52/1 -> To Workspace74/1
  Gateway Out43/1 -> To Workspace92/1
  Gateway Out45/1 -> To Workspace93/1
  Gateway Out50/1 -> To Workspace94/1
  Gateway Out53/1 -> To Workspace95/1
  Gateway Out40/1 -> To Workspace71/1
  bus_expand10/2(out7) -> pipeline40/1(d)
  bus_expand10/4(out5) -> pipeline97/1(d)
  bus_expand10/6(out3) -> pipeline101/1(d)
  bus_expand10/8(lsb_out1) -> pipeline103/1(d)
  bus_expand10/7(out2) -> pipeline102/1(d)
  bus_expand10/5(out4) -> pipeline100/1(d)
  bus_expand10/3(out6) -> pipeline47/1(d)
  bus_expand10/1(msb_out8) -> pipeline39/1(d)
  [pfb_dfloat_0] -> bus_expand10/1(bus_in)
  [pfb_dv] -> Counter3/2
  tvg_sel/1(in_tvg_sel) -> [tvg_sel]
  [tvg_sel] -> pipeline77/1(d)
  pfb_of/1(sim_out) -> Terminator9/1
  pipeline6/1(q) -> spectrum_counter/1(out_reg)
  pipeline7/1(q) -> pfb_of/1(out_reg)
  Constant29/1 -> sys_en/1(sim_1)
  sys_en/1(in_en) -> pipeline4/1(d)
  [sys_en] -> Counter/2
  Concat/1 -> pipeline75/1(d)
  [cwg_80_0] -> pipeline76/1(d)
  [pfb_of] -> pipeline7/1(d)
  [pfb_sync] -> Logical12/1
  [rst] -> Logical12/2
  Logical12/1 -> [local_rst]
  pipeline4/1(q) -> pipeline12/1(d)
  pipeline12/1(q) -> [sys_en]
  acc_cntrl/1(new_acc) -> [new_acc]
  [acc_len] -> acc_cntrl/1(acc_len)
  Delay14/1 -> Delay10/1
  Delay10/1 -> Delay35/1
  simple_bram_vacc/2(valid) -> pipeline26/1(d), spect_cnt/2
  spect_cnt/1 -> pipeline27/1(d)
  simple_bram_vacc/1(dout) -> pipeline25/1(d)
  Delay34/1 -> Delay6/1
  pipeline23/1(q) -> pipeline18/1(d)
  [new_acc] -> Gateway Out67/1, pipeline16/1(d)
  pipeline17/1(q) -> simple_bram_vacc/1(new_acc)
  pipeline18/1(q) -> simple_bram_vacc/2(din)
  pipeline16/1(q) -> spect_cnt/1
  Delay24/1 -> acc_cntrl/2(sync)
  [pfb_sync] -> pipeline54/1(d)
  cnt_rst/1(in_reg) -> pipeline19/1(d)
  Constant14/1 -> cnt_rst/1(sim_1)
  Constant16/1 -> acc_len/1(sim_1)
  acc_len/1(in_reg) -> pipeline13/1(d)
  pipeline19/1(q) -> Slice2/1
  Slice2/1 -> [cnt_rst]
  pipeline13/1(q) -> [acc_len]
  [new_acc] -> pipeline17/1(d)
  Gateway Out15/1 -> To Workspace16/1
  Gateway Out16/1 -> To Workspace17/1
  Gateway Out17/1 -> To Workspace18/1
  [pfb_r0] -> pipeline92/1(d)
  [pfb_i0] -> pipeline93/1(d)
  simple_bram_vacc1/2(valid) -> pipeline28/1(d)
  simple_bram_vacc1/1(dout) -> pipeline24/1(d)
  pipeline22/1(q) -> pipeline21/1(d)
  pipeline20/1(q) -> simple_bram_vacc1/1(new_acc)
  pipeline21/1(q) -> simple_bram_vacc1/2(din)
  [new_acc] -> pipeline20/1(d)
  Gateway Out27/1 -> To Workspace26/1
  [pfb_r1] -> pipeline85/1(d)
  [pfb_i1] -> pipeline86/1(d)
  simple_bram_vacc2/2(valid) -> pipeline32/1(d)
  simple_bram_vacc2/1(dout) -> pipeline31/1(d)
  pipeline30/1(q) -> pipeline29/1(d)
  pipeline14/1(q) -> simple_bram_vacc2/1(new_acc)
  pipeline29/1(q) -> simple_bram_vacc2/2(din)
  [new_acc] -> pipeline14/1(d)
  Gateway Out1/1 -> To Workspace4/1
  [pfb_r2] -> pipeline87/1(d)
  [pfb_i2] -> pipeline88/1(d)
  simple_bram_vacc3/2(valid) -> pipeline37/1(d)
  simple_bram_vacc3/1(dout) -> pipeline36/1(d)
  pipeline35/1(q) -> pipeline34/1(d)
  pipeline33/1(q) -> simple_bram_vacc3/1(new_acc)
  pipeline34/1(q) -> simple_bram_vacc3/2(din)
  [new_acc] -> pipeline33/1(d)
  Gateway Out57/1 -> To Workspace52/1
  [pfb_r3] -> pipeline89/1(d)
  [pfb_i3] -> pipeline91/1(d)
  pipeline27/1(q) -> [acc]
  [acc] -> Gateway Out15/1
  pipeline26/1(q) -> [acc_dv]
  pipeline25/1(q) -> [acc_d0]
  [acc_dv] -> Gateway Out17/1
  pipeline24/1(q) -> [acc_d1]
  pipeline31/1(q) -> [acc_d2]
  pipeline36/1(q) -> [acc_d3]
  ss_pfb_sq0/1(arm_out) -> Terminator4/1
  [acc_dv] -> pipeline42/1(d)
  [new_acc] -> pipeline43/1(d)
  edge_detect4/1 -> ss_pfb_sq0/3(trig)
  [acc_d1] -> pipeline46/1(d)
  [acc_dv] -> pipeline49/1(d)
  edge_detect7/1 -> ss_pfb_sq1/3(trig)
  [acc_d2] -> pipeline15/1(d)
  [acc_dv] -> pipeline48/1(d)
  edge_detect8/1 -> ss_pfb_sq2/3(trig)
  Logical/1 -> acc_cntrl/3(rst)
  [cnt_rst] -> Logical/2
  pipeline75/1(q) -> Mux/3
  pipeline76/1(q) -> Mux/2
  pipeline77/1(q) -> Mux/1
  pipeline79/1(q) -> pipeline5/1(d), edge_detect/1
  pipeline81/1(q) -> [pfb_dfloat_0]
  pipeline82/1(q) -> [pfb_dfloat_1]
  pipeline84/1(q) -> [pfb_of]
  pipeline95/1(q) -> Delay14/1
  [acc_d3] -> pipeline96/1(d)
  [acc_dv] -> pipeline98/1(d)
  edge_detect6/1 -> ss_pfb_sq3/3(trig)
  ss_pfb_sq1/1(arm_out) -> Terminator5/1
  ss_pfb_sq2/1(arm_out) -> Terminator6/1
  ss_pfb_sq3/1(arm_out) -> Terminator7/1
  pipeline39/1(q) -> [pfb_r0]
  pipeline40/1(q) -> [pfb_i0]
  pipeline47/1(q) -> [pfb_r1]
  pipeline97/1(q) -> [pfb_i1]
  pipeline100/1(q) -> [pfb_r2]
  pipeline101/1(q) -> [pfb_i2]
  pipeline102/1(q) -> [pfb_r3]
  pipeline103/1(q) -> [pfb_i3]
  pipeline80/1(q) -> [pfb_sync]
  pipeline83/1(q) -> [pfb_dv]
  Concat1/1 -> Concat/2
  pipeline28/1(q) -> Terminator8/1
  pipeline32/1(q) -> Terminator11/1
  pipeline37/1(q) -> Terminator12/1
  Delay25/1 -> pipeline23/1(d)
  Delay8/1 -> pipeline22/1(d)
  Delay7/1 -> pipeline30/1(d)
  Delay16/1 -> pipeline35/1(d)
  pipeline92/1(q) -> Delay15/1
  real_square/1 -> AddSub/1
  pipeline93/1(q) -> Delay5/1
  imag_square/1 -> AddSub/2
  Delay15/1 -> Delay17/1
  Delay17/1 -> Reinterpret17/1
  Delay5/1 -> Delay9/1
  Delay9/1 -> Reinterpret1/1
  real_square1/1 -> AddSub1/1
  imag_square1/1 -> AddSub1/2
  Delay3/1 -> Reinterpret18/1
  Delay11/1 -> Reinterpret8/1
  Delay1/1 -> Delay3/1
  Delay4/1 -> Delay11/1
  real_square2/1 -> AddSub2/1
  imag_square2/1 -> AddSub2/2
  Delay18/1 -> Reinterpret2/1
  Delay13/1 -> Reinterpret3/1
  Delay12/1 -> Delay18/1
  Delay19/1 -> Delay13/1
  real_square3/1 -> AddSub3/1
  imag_square3/1 -> AddSub3/2
  Delay22/1 -> Reinterpret4/1
  Delay21/1 -> Reinterpret5/1
  Delay20/1 -> Delay22/1
  Delay23/1 -> Delay21/1
  pipeline85/1(q) -> Delay1/1
  pipeline86/1(q) -> Delay4/1
  pipeline87/1(q) -> Delay12/1
  pipeline88/1(q) -> Delay19/1
  pipeline89/1(q) -> Delay20/1
  pipeline91/1(q) -> Delay23/1
  Reinterpret16/1 -> Gateway Out40/1
  Reinterpret9/1 -> Gateway Out43/1
  Reinterpret10/1 -> Gateway Out44/1
  Reinterpret11/1 -> Gateway Out45/1
  Reinterpret12/1 -> Gateway Out47/1
  Reinterpret13/1 -> Gateway Out50/1
  Reinterpret14/1 -> Gateway Out52/1
  Reinterpret15/1 -> Gateway Out53/1
  AddSub/1 -> Convert4/1
  AddSub1/1 -> Convert1/1
  AddSub2/1 -> Convert2/1
  AddSub3/1 -> Convert3/1
  [acc_d0] -> pipeline38/1(d)
  [pfb_dfloat_0] -> bus_expand1/1(bus_in)
  bus_expand1/1(msb_out8) -> Reinterpret16/1
  bus_expand1/2(out7) -> Reinterpret9/1
  bus_expand1/3(out6) -> Reinterpret10/1
  bus_expand1/4(out5) -> Reinterpret11/1
  bus_expand1/5(out4) -> Reinterpret12/1
  bus_expand1/6(out3) -> Reinterpret13/1
  bus_expand1/7(out2) -> Reinterpret14/1
  bus_expand1/8(lsb_out1) -> Reinterpret15/1
  pipeline54/1(q) -> pipeline95/1(d)
  wng0/1(bus_out) -> [wng0_tp0], pipeline78/1(d)
  bus_addsub2/1(dout) -> bus_expand4/1(bus_in), bus_convert6/1(din)
  Constant35/1 -> scale_wng0/1(sim_1)
  scale_wng0/1(in_scale) -> pipeline57/1(d), Gateway Out24/1
  bus_addsub1/1(dout) -> bus_convert2/1(din)
  bus_addsub3/1(dout) -> bus_convert4/1(din)
  Delay139/1 -> bus_addsub2/1(a)
  pipeline56/1(q) -> bus_mult1/2(b)
  pipeline78/1(q) -> bus_addsub1/1(a)
  pipeline94/1(q) -> [cwg_80_0]
  Delay2/1 -> bus_addsub2/2(b)
  bus_convert/1(dout) -> [cwg0_tp2], pipeline94/1(d)
  bus_convert2/1(dout) -> [wng0_sum], Delay139/1
  bus_convert4/1(dout) -> Delay2/1
  bus_convert6/1(dout) -> bus_expand6/1(bus_in), pipeline55/1(d)
  scale_out0/1(in_scale) -> pipeline56/1(d)
  Constant12/1 -> freq_cwg0/1(sim_1)
  freq_cwg0/1(in_frequency) -> pipeline45/1(d)
  Constant37/1 -> scale_cwg0/2(sim_2)
  Constant72/1 -> scale_cwg0/1(sim_1)
  Constant57/1 -> scale_out0/1(sim_1)
  Constant61/1 -> scale_out0/2(sim_2)
  scale_out0/2(in_dummy) -> Terminator/1
  pipeline45/1(q) -> cwg0/1(freq)
  scale_cwg0/1(in_scale) -> pipeline44/1(d), Gateway Out23/1
  Constant60/1 -> rpt_en_cwg0/2(sim_2)
  Constant62/1 -> rpt_length_cwg0/1(sim_1)
  rpt_en_cwg0/1(in_en) -> Convert25/1
  rpt_en_cwg0/2(in_dummy) -> Terminator57/1
  Constant70/1 -> rpt_en_cwg0/1(sim_1)
  cwg0/1(bus_out) -> pipeline90/1(d), [Bus_expand_cwg0]
  [mrst] -> pipeline11/1(d)
  Convert25/1 -> pipeline50/1(d)
  rpt_length_cwg0/1(in_cwg0_repeat_length) -> pipeline51/1(d)
  cwg0/3(rpt_rst) -> Terminator61/1
  Logical20/1 -> pipeline41/1(d)
  Constant23/1 -> man_rst_wng0/1(sim_1)
  man_rst_wng0/1(in_rst) -> [wng0_man_rst], Logical20/2
  [wng0_rst] -> Logical20/1
  pipeline55/1(q) -> bus_mult1/1(a), [cwg0_tp0]
  Gateway Out24/1 -> To Workspace24/1
  Constant103/1 -> scale_wng0/2(sim_2)
  Constant106/1 -> cwg0/6(en)
  pipeline90/1(q) -> bus_addsub3/1(a)
  cwg0/2(dvalid) -> Terminator28/1
  Gateway Out23/1 -> To Workspace29/1
  Constant5/1 -> [wng0_rst]
  Constant6/1 -> [mrst]
  [pfbin_d0] -> bus_expand5/1(bus_in)
  Gateway Out29/1 -> To Workspace30/1
  bus_expand5/1(msb_out8) -> Gateway Out29/1
  Gateway Out37/1 -> To Workspace32/1
  bus_expand5/8(lsb_out1) -> Gateway Out37/1
  Gateway Out36/1 -> To Workspace33/1
  bus_expand5/7(out2) -> Gateway Out36/1
  Gateway Out35/1 -> To Workspace34/1
  bus_expand5/6(out3) -> Gateway Out35/1
  Gateway Out34/1 -> To Workspace35/1
  bus_expand5/5(out4) -> Gateway Out34/1
  Gateway Out33/1 -> To Workspace36/1
  bus_expand5/4(out5) -> Gateway Out33/1
  Gateway Out32/1 -> To Workspace37/1
  bus_expand5/3(out6) -> Gateway Out32/1
  Gateway Out31/1 -> To Workspace38/1
  bus_expand5/2(out7) -> Gateway Out31/1
  Constant11/1 -> Terminator1/1
  Constant10/1 -> Terminator3/1
  bus_constant/1(dout) -> bus_addsub3/2(b)
  bus_constant1/1(dout) -> bus_addsub1/2(b)
  pipeline44/1(q) -> cwg0/2(scale)
  pipeline11/1(q) -> cwg0/3(rst)
  pipeline50/1(q) -> cwg0/4(repeat_en)
  pipeline51/1(q) -> cwg0/5(repeat_length)
  pipeline41/1(q) -> wng0/2(rst)
  pipeline57/1(q) -> wng0/1(scale_16_14)
  Delay6/1 -> Delay26/1
  [acc_d0] -> Gateway Out16/1
  [acc_d1] -> Gateway Out27/1
  [acc_d2] -> Gateway Out1/1
  [acc_d3] -> Gateway Out57/1
  pipeline42/1(q) -> ss_pfb_sq0/2(we)
  pipeline43/1(q) -> edge_detect4/1
  pipeline49/1(q) -> ss_pfb_sq1/2(we)
  pipeline52/1(q) -> edge_detect7/1
  pipeline48/1(q) -> ss_pfb_sq2/2(we)
  pipeline53/1(q) -> edge_detect8/1
  pipeline99/1(q) -> edge_detect6/1
  pipeline98/1(q) -> ss_pfb_sq3/2(we)
  Gateway Out6/1 -> To Workspace8/1
  Gateway Out7/1 -> To Workspace9/1
  Gateway Out10/1 -> To Workspace11/1
  Gateway Out11/1 -> To Workspace12/1
  pipeline46/1(q) -> Gateway Out7/1, ss_pfb_sq1/1(in_pfb2_0)
  pipeline15/1(q) -> Gateway Out10/1, ss_pfb_sq2/1(in_pfb2_0)
  pipeline96/1(q) -> Gateway Out11/1, ss_pfb_sq3/1(in_pfb2_0)
  Constant13/1 -> acc_scale/1(sim_1)
  acc_scale/1(in_reg) -> pipeline58/1(d)
  pipeline58/1(q) -> [acc_scale]
  [acc_scale] -> scale_float/3(scale)
  Reinterpret17/1 -> scale_float/1(real)
  Reinterpret1/1 -> scale_float/2(imag)
  scale_float/1(real_out) -> real_square/2, real_square/1
  scale_float/2(imag_out) -> imag_square/1, imag_square/2
  Delay35/1 -> Delay34/1
  Convert4/1 -> Gateway Out12/1, Delay25/1
  pipeline38/1(q) -> Gateway Out6/1, ss_pfb_sq0/1(in_pfb2_0)
  Gateway Out12/1 -> To Workspace13/1
  Convert1/1 -> Delay8/1
  Convert2/1 -> Delay7/1
  Convert3/1 -> Delay16/1
  Reinterpret18/1 -> scale_float1/1(real)
  Reinterpret8/1 -> scale_float1/2(imag)
  Reinterpret2/1 -> scale_float2/1(real)
  Reinterpret3/1 -> scale_float2/2(imag)
  scale_float2/1(real_out) -> real_square2/2, real_square2/1
  scale_float2/2(imag_out) -> imag_square2/1, imag_square2/2
  Reinterpret4/1 -> scale_float3/1(real)
  Reinterpret5/1 -> scale_float3/2(imag)
  scale_float3/1(real_out) -> real_square3/2, real_square3/1
  scale_float3/2(imag_out) -> imag_square3/1, imag_square3/2
  [acc_scale] -> scale_float1/3(scale)
  [acc_scale] -> scale_float2/3(scale)
  [acc_scale] -> scale_float3/3(scale)
  scale_float1/1(real_out) -> real_square1/2, real_square1/1
  scale_float1/2(imag_out) -> imag_square1/1, imag_square1/2
  Delay26/1 -> Delay24/1, Logical/1
  simple_bram_vacc_float1/1(dout) -> Terminator10/1
  simple_bram_vacc_float1/2(valid) -> Terminator13/1
  Constant15/1 -> simple_bram_vacc_float1/2(din)
  [new_acc] -> simple_bram_vacc_float1/1(new_acc)
  Gateway Out2/1 -> To Workspace5/1
  bus_expand2/1(msb_out8) -> Gateway Out2/1
  Gateway Out3/1 -> To Workspace6/1
  bus_expand2/2(out7) -> Gateway Out3/1
  Gateway Out4/1 -> To Workspace7/1
  Gateway Out5/1 -> To Workspace14/1
  bus_expand2/4(out5) -> Gateway Out5/1
  bus_expand2/3(out6) -> Gateway Out4/1
  Gateway Out13/1 -> To Workspace19/1
  Gateway Out14/1 -> To Workspace15/1
  bus_expand2/6(out3) -> Gateway Out14/1
  bus_expand2/5(out4) -> Gateway Out13/1
  Gateway Out19/1 -> To Workspace21/1
  Gateway Out20/1 -> To Workspace20/1
  bus_expand2/7(out2) -> Gateway Out19/1
  bus_expand2/8(lsb_out1) -> Gateway Out20/1
  [pfb_dfloat_0] -> float_to_fix/1(In1)
  [acc_scale] -> float_to_fix/2(In2)
  float_to_fix/1(Out1) -> [pfb_raw], bus_expand2/1(bus_in)
  bus_mult1/1(a*b) -> bus_expand3/1(bus_in), [cwg0_tp1], bus_convert/1(din)
  Gateway Out21/1 -> To Workspace2/1
  bus_expand3/1(msb_out8) -> Gateway Out21/1
  Gateway Out39/1 -> To Workspace22/1
  bus_expand3/8(lsb_out1) -> Gateway Out39/1
  Gateway Out38/1 -> To Workspace23/1
  bus_expand3/7(out2) -> Gateway Out38/1
  Gateway Out30/1 -> To Workspace25/1
  bus_expand3/6(out3) -> Gateway Out30/1
  Gateway Out28/1 -> To Workspace27/1
  bus_expand3/5(out4) -> Gateway Out28/1
  Gateway Out26/1 -> To Workspace28/1
  bus_expand3/4(out5) -> Gateway Out26/1
  Gateway Out25/1 -> To Workspace31/1
  bus_expand3/3(out6) -> Gateway Out25/1
  Gateway Out22/1 -> To Workspace39/1
  bus_expand3/2(out7) -> Gateway Out22/1
  Gateway Out42/1 -> To Workspace40/1
  bus_expand4/1(msb_out8) -> Gateway Out42/1
  Gateway Out58/1 -> To Workspace41/1
  bus_expand4/8(lsb_out1) -> Gateway Out58/1
  Gateway Out56/1 -> To Workspace43/1
  bus_expand4/7(out2) -> Gateway Out56/1
  Gateway Out55/1 -> To Workspace45/1
  bus_expand4/6(out3) -> Gateway Out55/1
  Gateway Out54/1 -> To Workspace46/1
  bus_expand4/5(out4) -> Gateway Out54/1
  Gateway Out49/1 -> To Workspace47/1
  bus_expand4/4(out5) -> Gateway Out49/1
  Gateway Out48/1 -> To Workspace48/1
  bus_expand4/3(out6) -> Gateway Out48/1
  Gateway Out46/1 -> To Workspace49/1
  bus_expand4/2(out7) -> Gateway Out46/1
  Gateway Out59/1 -> To Workspace50/1
  bus_expand6/1(msb_out8) -> Gateway Out59/1
  Gateway Out66/1 -> To Workspace51/1
  bus_expand6/8(lsb_out1) -> Gateway Out66/1
  Gateway Out65/1 -> To Workspace53/1
  bus_expand6/7(out2) -> Gateway Out65/1
  Gateway Out64/1 -> To Workspace54/1
  bus_expand6/6(out3) -> Gateway Out64/1
  Gateway Out63/1 -> To Workspace55/1
  bus_expand6/5(out4) -> Gateway Out63/1
  Gateway Out62/1 -> To Workspace56/1
  bus_expand6/4(out5) -> Gateway Out62/1
  Gateway Out61/1 -> To Workspace57/1
  bus_expand6/3(out6) -> Gateway Out61/1
  Gateway Out60/1 -> To Workspace58/1
  bus_expand6/2(out7) -> Gateway Out60/1
  Gateway Out67/1 -> To Workspace59/1
  ss_pfb_raw/1(arm_out) -> Terminator14/1
  [acc_dv] -> pipeline60/1(d)
  [new_acc] -> pipeline61/1(d)
  edge_detect9/1 -> ss_pfb_raw/7(trig)
  pipeline60/1(q) -> ss_pfb_raw/6(we)
  pipeline61/1(q) -> edge_detect9/1
  [acc_dv] -> pipeline63/1(d)
  [new_acc] -> pipeline64/1(d)
  pipeline64/1(q) -> edge_detect10/1
  [pfb_raw] -> bus_expand7/1(bus_in)
  pipeline65/1(q) -> ss_pfb_raw/2(in_i0)
  pipeline59/1(q) -> ss_pfb_raw/1(in_r0)
  pipeline66/1(q) -> ss_pfb_raw/3(in_r1)
  pipeline67/1(q) -> ss_pfb_raw/4(in_i1)
  bus_expand7/1(msb_out8) -> pipeline59/1(d)
  bus_expand7/2(out7) -> pipeline65/1(d)
  bus_expand7/3(out6) -> pipeline66/1(d)
  bus_expand7/4(out5) -> pipeline67/1(d)
  ss_pfb_raw1/1(arm_out) -> Terminator15/1
  edge_detect10/1 -> ss_pfb_raw1/6(trig)
  pipeline63/1(q) -> ss_pfb_raw1/5(we)
  bus_expand7/5(out4) -> pipeline70/1(d)
  bus_expand7/6(out3) -> pipeline69/1(d)
  bus_expand7/7(out2) -> pipeline68/1(d)
  bus_expand7/8(lsb_out1) -> pipeline62/1(d)
  pipeline62/1(q) -> ss_pfb_raw1/4(in_i3)
  pipeline68/1(q) -> ss_pfb_raw1/3(in_r3)
  pipeline69/1(q) -> ss_pfb_raw1/2(in_i2)
  pipeline70/1(q) -> ss_pfb_raw1/1(in_r2)
  pipeline71/1(q) -> ss_pfb_raw/5(in_acc)
  [acc] -> pipeline71/1(d)
  [new_acc] -> pipeline52/1(d)
  [new_acc] -> pipeline53/1(d)
  [new_acc] -> pipeline99/1(d)
